simulink model "external_mode_serial_am263px"  (R2024b)
settings: Solver=FixedStepAuto  StartTime=0.0  StopTime=15  RelTol=1e-3  EnableMultiTasking=on
settings Model: Creator=a0499511  LastModifiedBy=a1255762
settings OptimizationCC: EnhancedBackFolding=on  PassReuseOutputArgsAs=Individual arguments  ZeroExternalMemoryAtStartup=off  ZeroInternalMemoryAtStartup=off  MaxStackSize=64  OptimizeBlockOrder=speed  DifferentSizesBufferReuse=on  OptimizationCustomize=off  EfficientTunableParamExpr=on
settings DebuggingCC: ParameterTunabilityLossMsg=error
settings HardwareCC: ProdBitPerPointer=32  ProdBitPerSizeT=32  ProdBitPerPtrDiffT=32  ProdLargestAtomicInteger=Long  ProdLargestAtomicFloat=Double  ProdWordSize=32  ProdLongLongMode=on  ProdHWDeviceType=ARM Compatible->ARM Cortex
settings SFSimCC: MATLABDynamicMemAlloc=off
settings RTWCC: Description=Embedded Coder  SystemTargetFile=ert.tlc  HardwareBoard=AM263PX LP  TemplateMakefile=ert_default_tmf  Toolchain=TI ARM Clang Compiler AM263Px | gmake  BuildConfiguration=Debug
settings CodeAppCC: ForceParamTrailComments=on  IgnoreCustomStorageClasses=off  MaxIdLength=256  OperatorAnnotations=on  SimulinkDataObjDesc=on  SFDataObjDesc=on  InsertBlockDesc=on
settings ERTTargetCC: SupportContinuousTime=on  ExtModeTransport=3  ExtModeStaticAlloc=on  ExtModeMexFile=ext_xcp  ExtModeIntrfLevel=Level2 - Open  InlinedParameterPlacement=Hierarchical  ERTCustomFileTemplate=AM263x_codertarget_file_process.tlc
settings ConfigComp: CovBoundaryAbsTol=1.0e-5

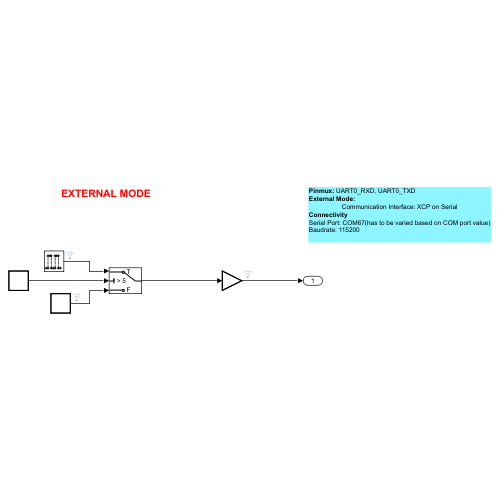
[thumbnail: Simulink's stored preview of the top-level diagram]
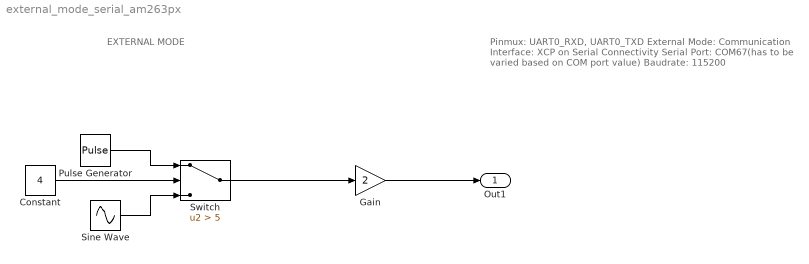
== system external_mode_serial_am263px ==
  note: EXTERNAL MODE
  note: Pinmux: UART0_RXD, UART0_TXD
      | External Mode:
      | Communication Interface: XCP on Serial
      | Connectivity
      | Serial Port: COM67(has to be varied based on COM port value)
      | Baudrate: 115200
  Constant : Constant  Value=4
  Gain : Gain  Gain=2
  Pulse Generator : DiscretePulseGenerator  Amplitude=10  Period=20  PulseWidth=15
  Sine Wave : Sin  Amplitude=10  Frequency=0.5  SampleTime=0
  Switch : Switch  Criteria=u2 > Threshold  Threshold=5  SaturateOnIntegerOverflow=off  InputSameDT=off
  Out1 : Outport
  wires:
  Gain/1 -> Out1/1
  Switch/1 -> Gain/1
  Constant/1 -> Switch/2
  Sine Wave/1 -> Switch/3
  Pulse Generator/1 -> Switch/1
